simulink model "r2_c8n856m1k_vacc"  (R2013a)
settings: Solver=FixedStepDiscrete  SolverMode=SingleTasking  StartTime=0.0  StopTime=10000  RelTol=1e-3
undefined symbols (not defined in the file): Down, Up
libraries: xbsIndex_r4 x32309, casper_library_misc x83, casper_library_delays x13, casper_library_communications x8, xbsReplacements_r4 x3, xps_library x2
(part 51 of 251)

== system r2_c8n856m1k_vacc/pfb/pfb_fir_generic/pfb_fir_taps/ddata0 ==
  din : Inport
  en : Inport  Port=2
  del{1..96} (96 alike) : xbsIndex_r4/Delay  rst=off  infoeditControl=Selection of Reset will increase slice count due to use of real FFs and instead of SRLs  en=on  latency=2  dbl_ovrd=off  reg_retiming=on  block_type=delay
  din_expand : Subsystem  AncestorBlock=casper_library_flow_control/bus_expand  mask(bus_expand){mode=divisions of arbitrary size, outputNum=1, outputWidth=[10  10  10  10  10  10  10  10  10  10  10  10  10  10  10  10  10  10  10  10  10  10  10  10  10  10  10  10  10  10  10  10  10  10  10  10  10  10  10  10  10  10  10  10  10  10  10  10  10  10  10  10  10  10  10  10  10  10  10  10  10  10  10  10  10  10  10  10  10  10  10  10  10  10  10  10  10  10  10  10  10  10  10  10  10  10  10  10  10  10  10  10  10  10  10  10], outputBinaryPt=[0  0  0  0  0  0  0  0  0  0  0  0  0  0  0  0  0  0  0  0  0  0  0  0  0  0  0  0  0  0  0  0  0  0  0  0  0  0  0  0  0  0  0  0  0  0  0  0  0  0  0  0  0  0  0  0  0  0  0  0  0  0  0  0  0  0  0  0  0  0  0  0  0  0  0  0  0  0  0  0  0  0  0  0  0  0  0  0  0  0  0  0  0  0  0  0], outputArithmeticType=[0  0  0  0  0  0  0  0  0  0  0  0  0  0  0  0  0  0  0  0  0  0  0  0  0  0  0  0  0  0  0  0  0  0  0  0  0  0  0  0  0  0  0  0  0  0  0  0  0  0  0  0  0  0  0  0  0  0  0  0  0  0  0  0  0  0  0  0  0  0  0  0  0  0  0  0  0  0  0  0  0  0  0  0  0  0  0  0  0  0  0  0  0  0  0  0], show_format=on, outputToWorkspace=off, variablePrefix=, outputToModelAsWell=on}
      mask prompts: outputNum "Number of outputs:"; outputWidth "Output width [msb ... lsb]:"; outputBinaryPt "Output binary point position [msb ... lsb]:"; outputArithmeticType "Output arithmetic type (ufix=0, fix=1, bool=2) [msb ... lsb]:"; show_format "Print format string?"; outputToWorkspace "Output to workspace?"; variablePrefix "Variable name prefix:"; outputToModelAsWell "Output to model as well?"
      mask help:
      | Expands a 'bus' made using a bus_create or Xilinx
      | concat block.
      | The input will be divided into the specified number
      | of outputs with the specified arithmetic types.
      | Divisions are made from most significant bit.
      mask init: same as r2_c8n856m1k_vacc/bus_expand15
      settings: AttributesFormatString=96 outputs: uf10.0,uf10.0,uf10.0,uf10.0,uf10.0,uf10.0,uf10.0,uf10.0,uf10.0,uf10.0,uf10.0,uf10.0,uf10.0,uf10.0,uf10.0,uf10.0,uf10.0,uf10.0,uf10.0,uf10.0,uf10.0,uf10.0,uf10.0,uf10.0,uf10.0,uf10.0,uf10.0,uf10.0,uf10.0,uf10.0,uf10.0,uf10.0,uf10.0,uf10.0,uf10.0,uf10.0,uf10.0,uf10.0,uf10.0,uf10.0,uf10.0,uf10.0,uf10.0,uf10.0,uf10.0,uf10.0,uf10.0,uf10.0,uf10.0,uf10.0,uf10.0,uf10.0,uf10.0,uf10.0,uf10.0,uf10.0,uf10.0,uf10.0,uf10.0,uf10.0,uf10.0,uf10.0,uf10.0,uf10.0,uf10.0,uf10.0,uf10.0,uf10.0,uf10.0,uf10.0,uf10.0,uf10.0,uf10.0,uf10.0,uf10.0,uf10.0,uf10.0,uf10.0,uf10.0,uf10.0,uf10.0,uf10.0,uf10.0,uf10.0,uf10.0,uf10.0,uf10.0,uf10.0,uf10.0,uf10.0,uf10.0,uf10.0,uf10.0,uf10.0,uf10.0,uf10.0,
      mask TypeOptions mode: divisions of equal size | divisions of arbitrary size
      mask Callback mode: bus_expand_callback();
      mask Callback outputWidth: bus_expand_callback();
      mask Callback outputToWorkspace: bus_expand_callback();
  dout_compress : Subsystem  AncestorBlock=casper_library_flow_control/bus_create  mask(bus_create){inputNum=96}
      mask prompts: inputNum "Number of inputs:"
      mask init:
      | bus_create_init(gcb,...
      |     'inputNum', inputNum);
  en_expand : Subsystem  AncestorBlock=casper_library_flow_control/bus_expand  mask(bus_expand){mode=divisions of equal size, outputNum=96, outputWidth=1, outputBinaryPt=0, outputArithmeticType=2, show_format=on, outputToWorkspace=off, variablePrefix=, outputToModelAsWell=on}
      mask prompts: outputNum "Number of outputs:"; outputWidth "Output width:"; outputBinaryPt "Output binary point position:"; outputArithmeticType "Output arithmetic type (ufix=0, fix=1, bool=2):"; show_format "Print format string?"; outputToWorkspace "Output to workspace?"; variablePrefix "Variable name prefix:"; outputToModelAsWell "Output to model as well?"
      mask help:
      | Expands a 'bus' made using a bus_create or Xilinx
      | concat block.
      | The input will be divided into the specified number
      | of outputs with the specified arithmetic types.
      | Divisions are made from most significant bit.
      mask init: same as r2_c8n856m1k_vacc/bus_expand15
      settings: AttributesFormatString=96 outputs: b,b,b,b,b,b,b,b,b,b,b,b,b,b,b,b,b,b,b,b,b,b,b,b,b,b,b,b,b,b,b,b,b,b,b,b,b,b,b,b,b,b,b,b,b,b,b,b,b,b,b,b,b,b,b,b,b,b,b,b,b,b,b,b,b,b,b,b,b,b,b,b,b,b,b,b,b,b,b,b,b,b,b,b,b,b,b,b,b,b,b,b,b,b,b,b,
      mask TypeOptions mode: divisions of equal size | divisions of arbitrary size
      mask Callback mode: bus_expand_callback();
      mask Callback outputWidth: bus_expand_callback();
      mask Callback outputToWorkspace: bus_expand_callback();
  dout : Outport
  wires:
  din/1 -> din_expand/1(bus_in)
  en/1 -> en_expand/1(bus_in)
  din_expand/1(msb_out96) -> del1/1
  en_expand/1(msb_out96) -> del1/2
  din_expand/2(out95) -> del2/1
  en_expand/2(out95) -> del2/2
  din_expand/3(out94) -> del3/1
  en_expand/3(out94) -> del3/2
  din_expand/4(out93) -> del4/1
  en_expand/4(out93) -> del4/2
  din_expand/5(out92) -> del5/1
  en_expand/5(out92) -> del5/2
  din_expand/6(out91) -> del6/1
  en_expand/6(out91) -> del6/2
  din_expand/7(out90) -> del7/1
  en_expand/7(out90) -> del7/2
  din_expand/8(out89) -> del8/1
  en_expand/8(out89) -> del8/2
  din_expand/9(out88) -> del9/1
  en_expand/9(out88) -> del9/2
  din_expand/10(out87) -> del10/1
  en_expand/10(out87) -> del10/2
  din_expand/11(out86) -> del11/1
  en_expand/11(out86) -> del11/2
  din_expand/12(out85) -> del12/1
  en_expand/12(out85) -> del12/2
  din_expand/13(out84) -> del13/1
  en_expand/13(out84) -> del13/2
  din_expand/14(out83) -> del14/1
  en_expand/14(out83) -> del14/2
  din_expand/15(out82) -> del15/1
  en_expand/15(out82) -> del15/2
  din_expand/16(out81) -> del16/1
  en_expand/16(out81) -> del16/2
  din_expand/17(out80) -> del17/1
  en_expand/17(out80) -> del17/2
  din_expand/18(out79) -> del18/1
  en_expand/18(out79) -> del18/2
  din_expand/19(out78) -> del19/1
  en_expand/19(out78) -> del19/2
  din_expand/20(out77) -> del20/1
  en_expand/20(out77) -> del20/2
  din_expand/21(out76) -> del21/1
  en_expand/21(out76) -> del21/2
  din_expand/22(out75) -> del22/1
  en_expand/22(out75) -> del22/2
  din_expand/23(out74) -> del23/1
  en_expand/23(out74) -> del23/2
  din_expand/24(out73) -> del24/1
  en_expand/24(out73) -> del24/2
  din_expand/25(out72) -> del25/1
  en_expand/25(out72) -> del25/2
  din_expand/26(out71) -> del26/1
  en_expand/26(out71) -> del26/2
  din_expand/27(out70) -> del27/1
  en_expand/27(out70) -> del27/2
  din_expand/28(out69) -> del28/1
  en_expand/28(out69) -> del28/2
  din_expand/29(out68) -> del29/1
  en_expand/29(out68) -> del29/2
  din_expand/30(out67) -> del30/1
  en_expand/30(out67) -> del30/2
  din_expand/31(out66) -> del31/1
  en_expand/31(out66) -> del31/2
  din_expand/32(out65) -> del32/1
  en_expand/32(out65) -> del32/2
  din_expand/33(out64) -> del33/1
  en_expand/33(out64) -> del33/2
  din_expand/34(out63) -> del34/1
  en_expand/34(out63) -> del34/2
  din_expand/35(out62) -> del35/1
  en_expand/35(out62) -> del35/2
  din_expand/36(out61) -> del36/1
  en_expand/36(out61) -> del36/2
  din_expand/37(out60) -> del37/1
  en_expand/37(out60) -> del37/2
  din_expand/38(out59) -> del38/1
  en_expand/38(out59) -> del38/2
  din_expand/39(out58) -> del39/1
  en_expand/39(out58) -> del39/2
  din_expand/40(out57) -> del40/1
  en_expand/40(out57) -> del40/2
  din_expand/41(out56) -> del41/1
  en_expand/41(out56) -> del41/2
  din_expand/42(out55) -> del42/1
  en_expand/42(out55) -> del42/2
  din_expand/43(out54) -> del43/1
  en_expand/43(out54) -> del43/2
  din_expand/44(out53) -> del44/1
  en_expand/44(out53) -> del44/2
  din_expand/45(out52) -> del45/1
  en_expand/45(out52) -> del45/2
  din_expand/46(out51) -> del46/1
  en_expand/46(out51) -> del46/2
  din_expand/47(out50) -> del47/1
  en_expand/47(out50) -> del47/2
  din_expand/48(out49) -> del48/1
  en_expand/48(out49) -> del48/2
  din_expand/49(out48) -> del49/1
  en_expand/49(out48) -> del49/2
  din_expand/50(out47) -> del50/1
  en_expand/50(out47) -> del50/2
  din_expand/51(out46) -> del51/1
  en_expand/51(out46) -> del51/2
  din_expand/52(out45) -> del52/1
  en_expand/52(out45) -> del52/2
  din_expand/53(out44) -> del53/1
  en_expand/53(out44) -> del53/2
  din_expand/54(out43) -> del54/1
  en_expand/54(out43) -> del54/2
  din_expand/55(out42) -> del55/1
  en_expand/55(out42) -> del55/2
  din_expand/56(out41) -> del56/1
  en_expand/56(out41) -> del56/2
  din_expand/57(out40) -> del57/1
  en_expand/57(out40) -> del57/2
  din_expand/58(out39) -> del58/1
  en_expand/58(out39) -> del58/2
  din_expand/59(out38) -> del59/1
  en_expand/59(out38) -> del59/2
  din_expand/60(out37) -> del60/1
  en_expand/60(out37) -> del60/2
  din_expand/61(out36) -> del61/1
  en_expand/61(out36) -> del61/2
  din_expand/62(out35) -> del62/1
  en_expand/62(out35) -> del62/2
  din_expand/63(out34) -> del63/1
  en_expand/63(out34) -> del63/2
  din_expand/64(out33) -> del64/1
  en_expand/64(out33) -> del64/2
  din_expand/65(out32) -> del65/1
  en_expand/65(out32) -> del65/2
  din_expand/66(out31) -> del66/1
  en_expand/66(out31) -> del66/2
  din_expand/67(out30) -> del67/1
  en_expand/67(out30) -> del67/2
  din_expand/68(out29) -> del68/1
  en_expand/68(out29) -> del68/2
  din_expand/69(out28) -> del69/1
  en_expand/69(out28) -> del69/2
  din_expand/70(out27) -> del70/1
  en_expand/70(out27) -> del70/2
  din_expand/71(out26) -> del71/1
  en_expand/71(out26) -> del71/2
  din_expand/72(out25) -> del72/1
  en_expand/72(out25) -> del72/2
  din_expand/73(out24) -> del73/1
  en_expand/73(out24) -> del73/2
  din_expand/74(out23) -> del74/1
  en_expand/74(out23) -> del74/2
  din_expand/75(out22) -> del75/1
  en_expand/75(out22) -> del75/2
  din_expand/76(out21) -> del76/1
  en_expand/76(out21) -> del76/2
  din_expand/77(out20) -> del77/1
  en_expand/77(out20) -> del77/2
  din_expand/78(out19) -> del78/1
  en_expand/78(out19) -> del78/2
  din_expand/79(out18) -> del79/1
  en_expand/79(out18) -> del79/2
  din_expand/80(out17) -> del80/1
  en_expand/80(out17) -> del80/2
  din_expand/81(out16) -> del81/1
  en_expand/81(out16) -> del81/2
  din_expand/82(out15) -> del82/1
  en_expand/82(out15) -> del82/2
  din_expand/83(out14) -> del83/1
  en_expand/83(out14) -> del83/2
  din_expand/84(out13) -> del84/1
  en_expand/84(out13) -> del84/2
  din_expand/85(out12) -> del85/1
  en_expand/85(out12) -> del85/2
  din_expand/86(out11) -> del86/1
  en_expand/86(out11) -> del86/2
  din_expand/87(out10) -> del87/1
  en_expand/87(out10) -> del87/2
  din_expand/88(out9) -> del88/1
  en_expand/88(out9) -> del88/2
  din_expand/89(out8) -> del89/1
  en_expand/89(out8) -> del89/2
  din_expand/90(out7) -> del90/1
  en_expand/90(out7) -> del90/2
  din_expand/91(out6) -> del91/1
  en_expand/91(out6) -> del91/2
  din_expand/92(out5) -> del92/1
  en_expand/92(out5) -> del92/2
  din_expand/93(out4) -> del93/1
  en_expand/93(out4) -> del93/2
  din_expand/94(out3) -> del94/1
  en_expand/94(out3) -> del94/2
  din_expand/95(out2) -> del95/1
  en_expand/95(out2) -> del95/2
  din_expand/96(lsb_out1) -> del96/1
  en_expand/96(lsb_out1) -> del96/2
  del1/1 -> dout_compress/1(in1)
  del2/1 -> dout_compress/2(in2)
  del3/1 -> dout_compress/3(in3)
  del4/1 -> dout_compress/4(in4)
  del5/1 -> dout_compress/5(in5)
  del6/1 -> dout_compress/6(in6)
  del7/1 -> dout_compress/7(in7)
  del8/1 -> dout_compress/8(in8)
  del9/1 -> dout_compress/9(in9)
  del10/1 -> dout_compress/10(in10)
  del11/1 -> dout_compress/11(in11)
  del12/1 -> dout_compress/12(in12)
  del13/1 -> dout_compress/13(in13)
  del14/1 -> dout_compress/14(in14)
  del15/1 -> dout_compress/15(in15)
  del16/1 -> dout_compress/16(in16)
  del17/1 -> dout_compress/17(in17)
  del18/1 -> dout_compress/18(in18)
  del19/1 -> dout_compress/19(in19)
  del20/1 -> dout_compress/20(in20)
  del21/1 -> dout_compress/21(in21)
  del22/1 -> dout_compress/22(in22)
  del23/1 -> dout_compress/23(in23)
  del24/1 -> dout_compress/24(in24)
  del25/1 -> dout_compress/25(in25)
  del26/1 -> dout_compress/26(in26)
  del27/1 -> dout_compress/27(in27)
  del28/1 -> dout_compress/28(in28)
  del29/1 -> dout_compress/29(in29)
  del30/1 -> dout_compress/30(in30)
  del31/1 -> dout_compress/31(in31)
  del32/1 -> dout_compress/32(in32)
  del33/1 -> dout_compress/33(in33)
  del34/1 -> dout_compress/34(in34)
  del35/1 -> dout_compress/35(in35)
  del36/1 -> dout_compress/36(in36)
  del37/1 -> dout_compress/37(in37)
  del38/1 -> dout_compress/38(in38)
  del39/1 -> dout_compress/39(in39)
  del40/1 -> dout_compress/40(in40)
  del41/1 -> dout_compress/41(in41)
  del42/1 -> dout_compress/42(in42)
  del43/1 -> dout_compress/43(in43)
  del44/1 -> dout_compress/44(in44)
  del45/1 -> dout_compress/45(in45)
  del46/1 -> dout_compress/46(in46)
  del47/1 -> dout_compress/47(in47)
  del48/1 -> dout_compress/48(in48)
  del49/1 -> dout_compress/49(in49)
  del50/1 -> dout_compress/50(in50)
  del51/1 -> dout_compress/51(in51)
  del52/1 -> dout_compress/52(in52)
  del53/1 -> dout_compress/53(in53)
  del54/1 -> dout_compress/54(in54)
  del55/1 -> dout_compress/55(in55)
  del56/1 -> dout_compress/56(in56)
  del57/1 -> dout_compress/57(in57)
  del58/1 -> dout_compress/58(in58)
  del59/1 -> dout_compress/59(in59)
  del60/1 -> dout_compress/60(in60)
  del61/1 -> dout_compress/61(in61)
  del62/1 -> dout_compress/62(in62)
  del63/1 -> dout_compress/63(in63)
  del64/1 -> dout_compress/64(in64)
  del65/1 -> dout_compress/65(in65)
  del66/1 -> dout_compress/66(in66)
  del67/1 -> dout_compress/67(in67)
  del68/1 -> dout_compress/68(in68)
  del69/1 -> dout_compress/69(in69)
  del70/1 -> dout_compress/70(in70)
  del71/1 -> dout_compress/71(in71)
  del72/1 -> dout_compress/72(in72)
  del73/1 -> dout_compress/73(in73)
  del74/1 -> dout_compress/74(in74)
  del75/1 -> dout_compress/75(in75)
  del76/1 -> dout_compress/76(in76)
  del77/1 -> dout_compress/77(in77)
  del78/1 -> dout_compress/78(in78)
  del79/1 -> dout_compress/79(in79)
  del80/1 -> dout_compress/80(in80)
  del81/1 -> dout_compress/81(in81)
  del82/1 -> dout_compress/82(in82)
  del83/1 -> dout_compress/83(in83)
  del84/1 -> dout_compress/84(in84)
  del85/1 -> dout_compress/85(in85)
  del86/1 -> dout_compress/86(in86)
  del87/1 -> dout_compress/87(in87)
  del88/1 -> dout_compress/88(in88)
  del89/1 -> dout_compress/89(in89)
  del90/1 -> dout_compress/90(in90)
  del91/1 -> dout_compress/91(in91)
  del92/1 -> dout_compress/92(in92)
  del93/1 -> dout_compress/93(in93)
  del94/1 -> dout_compress/94(in94)
  del95/1 -> dout_compress/95(in95)
  del96/1 -> dout_compress/96(in96)
  dout_compress/1(bus_out) -> dout/1

== system r2_c8n856m1k_vacc/pfb/pfb_fir_generic/pfb_fir_taps/ddata1 ==
  din : Inport
  en : Inport  Port=2
  del{1..112} (112 alike) : xbsIndex_r4/Delay  rst=off  infoeditControl=Selection of Reset will increase slice count due to use of real FFs and instead of SRLs  en=on  latency=2  dbl_ovrd=off  reg_retiming=on  block_type=delay
  din_expand : Subsystem  AncestorBlock=casper_library_flow_control/bus_expand  mask(bus_expand){mode=divisions of arbitrary size, outputNum=1, outputWidth=[10  10  10  10  10  10  10  10  10  10  10  10  10  10  10  10  10  10  10  10  10  10  10  10  10  10  10  10  10  10  10  10  10  10  10  10  10  10  10  10  10  10  10  10  10  10  10  10  10  10  10  10  10  10  10  10  10  10  10  10  10  10  10  10  10  10  10  10  10  10  10  10  10  10  10  10  10  10  10  10  10  10  10  10  10  10  10  10  10  10  10  10  10  10  10  10  10  10  10  10  10  10  10  10  10  10  10  10  10  10  10  10], outputBinaryPt=[0  0  0  0  0  0  0  0  0  0  0  0  0  0  0  0  0  0  0  0  0  0  0  0  0  0  0  0  0  0  0  0  0  0  0  0  0  0  0  0  0  0  0  0  0  0  0  0  0  0  0  0  0  0  0  0  0  0  0  0  0  0  0  0  0  0  0  0  0  0  0  0  0  0  0  0  0  0  0  0  0  0  0  0  0  0  0  0  0  0  0  0  0  0  0  0  0  0  0  0  0  0  0  0  0  0  0  0  0  0  0  0], outputArithmeticType=[0  0  0  0  0  0  0  0  0  0  0  0  0  0  0  0  0  0  0  0  0  0  0  0  0  0  0  0  0  0  0  0  0  0  0  0  0  0  0  0  0  0  0  0  0  0  0  0  0  0  0  0  0  0  0  0  0  0  0  0  0  0  0  0  0  0  0  0  0  0  0  0  0  0  0  0  0  0  0  0  0  0  0  0  0  0  0  0  0  0  0  0  0  0  0  0  0  0  0  0  0  0  0  0  0  0  0  0  0  0  0  0], show_format=on, outputToWorkspace=off, variablePrefix=, outputToModelAsWell=on}
      mask prompts: outputNum "Number of outputs:"; outputWidth "Output width [msb ... lsb]:"; outputBinaryPt "Output binary point position [msb ... lsb]:"; outputArithmeticType "Output arithmetic type (ufix=0, fix=1, bool=2) [msb ... lsb]:"; show_format "Print format string?"; outputToWorkspace "Output to workspace?"; variablePrefix "Variable name prefix:"; outputToModelAsWell "Output to model as well?"
      mask help:
      | Expands a 'bus' made using a bus_create or Xilinx
      | concat block.
      | The input will be divided into the specified number
      | of outputs with the specified arithmetic types.
      | Divisions are made from most significant bit.
      mask init: same as r2_c8n856m1k_vacc/bus_expand15
      settings: AttributesFormatString=112 outputs: uf10.0,uf10.0,uf10.0,uf10.0,uf10.0,uf10.0,uf10.0,uf10.0,uf10.0,uf10.0,uf10.0,uf10.0,uf10.0,uf10.0,uf10.0,uf10.0,uf10.0,uf10.0,uf10.0,uf10.0,uf10.0,uf10.0,uf10.0,uf10.0,uf10.0,uf10.0,uf10.0,uf10.0,uf10.0,uf10.0,uf10.0,uf10.0,uf10.0,uf10.0,uf10.0,uf10.0,uf10.0,uf10.0,uf10.0,uf10.0,uf10.0,uf10.0,uf10.0,uf10.0,uf10.0,uf10.0,uf10.0,uf10.0,uf10.0,uf10.0,uf10.0,uf10.0,uf10.0,uf10.0,uf10.0,uf10.0,uf10.0,uf10.0,uf10.0,uf10.0,uf10.0,uf10.0,uf10.0,uf10.0,uf10.0,uf10.0,uf10.0,uf10.0,uf10.0,uf10.0,uf10.0,uf10.0,uf10.0,uf10.0,uf10.0,uf10.0,uf10.0,uf10.0,uf10.0,uf10.0,uf10.0,uf10.0,uf10.0,uf10.0,uf10.0,uf10.0,uf10.0,uf10.0,uf10.0,uf10.0,uf10.0,uf10.0,uf10.0,uf10.0,uf10.0,uf10.0,uf10.0,uf10.0,uf10.0,uf10.0,uf10.0,uf10.0,uf10.0,uf10.0,uf10.0,uf10.0,uf10.0,uf10.0,uf10.0,uf10.0,uf10.0,uf10.0,
      mask TypeOptions mode: divisions of equal size | divisions of arbitrary size
      mask Callback mode: bus_expand_callback();
      mask Callback outputWidth: bus_expand_callback();
      mask Callback outputToWorkspace: bus_expand_callback();
  dout_compress : Subsystem  AncestorBlock=casper_library_flow_control/bus_create  mask(bus_create){inputNum=112}
      mask prompts: inputNum "Number of inputs:"
      mask init:
      | bus_create_init(gcb,...
      |     'inputNum', inputNum);
  en_expand : Subsystem  AncestorBlock=casper_library_flow_control/bus_expand  mask(bus_expand){mode=divisions of equal size, outputNum=112, outputWidth=1, outputBinaryPt=0, outputArithmeticType=2, show_format=on, outputToWorkspace=off, variablePrefix=, outputToModelAsWell=on}
      mask prompts: outputNum "Number of outputs:"; outputWidth "Output width:"; outputBinaryPt "Output binary point position:"; outputArithmeticType "Output arithmetic type (ufix=0, fix=1, bool=2):"; show_format "Print format string?"; outputToWorkspace "Output to workspace?"; variablePrefix "Variable name prefix:"; outputToModelAsWell "Output to model as well?"
      mask help:
      | Expands a 'bus' made using a bus_create or Xilinx
      | concat block.
      | The input will be divided into the specified number
      | of outputs with the specified arithmetic types.
      | Divisions are made from most significant bit.
      mask init: same as r2_c8n856m1k_vacc/bus_expand15
      settings: AttributesFormatString=112 outputs: b,b,b,b,b,b,b,b,b,b,b,b,b,b,b,b,b,b,b,b,b,b,b,b,b,b,b,b,b,b,b,b,b,b,b,b,b,b,b,b,b,b,b,b,b,b,b,b,b,b,b,b,b,b,b,b,b,b,b,b,b,b,b,b,b,b,b,b,b,b,b,b,b,b,b,b,b,b,b,b,b,b,b,b,b,b,b,b,b,b,b,b,b,b,b,b,b,b,b,b,b,b,b,b,b,b,b,b,b,b,b,b,
      mask TypeOptions mode: divisions of equal size | divisions of arbitrary size
      mask Callback mode: bus_expand_callback();
      mask Callback outputWidth: bus_expand_callback();
      mask Callback outputToWorkspace: bus_expand_callback();
  dout : Outport
  wires:
  din/1 -> din_expand/1(bus_in)
  en/1 -> en_expand/1(bus_in)
  din_expand/1(msb_out112) -> del1/1
  en_expand/1(msb_out112) -> del1/2
  din_expand/2(out111) -> del2/1
  en_expand/2(out111) -> del2/2
  din_expand/3(out110) -> del3/1
  en_expand/3(out110) -> del3/2
  din_expand/4(out109) -> del4/1
  en_expand/4(out109) -> del4/2
  din_expand/5(out108) -> del5/1
  en_expand/5(out108) -> del5/2
  din_expand/6(out107) -> del6/1
  en_expand/6(out107) -> del6/2
  din_expand/7(out106) -> del7/1
  en_expand/7(out106) -> del7/2
  din_expand/8(out105) -> del8/1
  en_expand/8(out105) -> del8/2
  din_expand/9(out104) -> del9/1
  en_expand/9(out104) -> del9/2
  din_expand/10(out103) -> del10/1
  en_expand/10(out103) -> del10/2
  din_expand/11(out102) -> del11/1
  en_expand/11(out102) -> del11/2
  din_expand/12(out101) -> del12/1
  en_expand/12(out101) -> del12/2
  din_expand/13(out100) -> del13/1
  en_expand/13(out100) -> del13/2
  din_expand/14(out99) -> del14/1
  en_expand/14(out99) -> del14/2
  din_expand/15(out98) -> del15/1
  en_expand/15(out98) -> del15/2
  din_expand/16(out97) -> del16/1
  en_expand/16(out97) -> del16/2
  din_expand/17(out96) -> del17/1
  en_expand/17(out96) -> del17/2
  din_expand/18(out95) -> del18/1
  en_expand/18(out95) -> del18/2
  din_expand/19(out94) -> del19/1
  en_expand/19(out94) -> del19/2
  din_expand/20(out93) -> del20/1
  en_expand/20(out93) -> del20/2
  din_expand/21(out92) -> del21/1
  en_expand/21(out92) -> del21/2
  din_expand/22(out91) -> del22/1
  en_expand/22(out91) -> del22/2
  din_expand/23(out90) -> del23/1
  en_expand/23(out90) -> del23/2
  din_expand/24(out89) -> del24/1
  en_expand/24(out89) -> del24/2
  din_expand/25(out88) -> del25/1
  en_expand/25(out88) -> del25/2
  din_expand/26(out87) -> del26/1
  en_expand/26(out87) -> del26/2
  din_expand/27(out86) -> del27/1
  en_expand/27(out86) -> del27/2
  din_expand/28(out85) -> del28/1
  en_expand/28(out85) -> del28/2
  din_expand/29(out84) -> del29/1
  en_expand/29(out84) -> del29/2
  din_expand/30(out83) -> del30/1
  en_expand/30(out83) -> del30/2
  din_expand/31(out82) -> del31/1
  en_expand/31(out82) -> del31/2
  din_expand/32(out81) -> del32/1
  en_expand/32(out81) -> del32/2
  din_expand/33(out80) -> del33/1
  en_expand/33(out80) -> del33/2
  din_expand/34(out79) -> del34/1
  en_expand/34(out79) -> del34/2
  din_expand/35(out78) -> del35/1
  en_expand/35(out78) -> del35/2
  din_expand/36(out77) -> del36/1
  en_expand/36(out77) -> del36/2
  din_expand/37(out76) -> del37/1
  en_expand/37(out76) -> del37/2
  din_expand/38(out75) -> del38/1
  en_expand/38(out75) -> del38/2
  din_expand/39(out74) -> del39/1
  en_expand/39(out74) -> del39/2
  din_expand/40(out73) -> del40/1
  en_expand/40(out73) -> del40/2
  din_expand/41(out72) -> del41/1
  en_expand/41(out72) -> del41/2
  din_expand/42(out71) -> del42/1
  en_expand/42(out71) -> del42/2
  din_expand/43(out70) -> del43/1
  en_expand/43(out70) -> del43/2
  din_expand/44(out69) -> del44/1
  en_expand/44(out69) -> del44/2
  din_expand/45(out68) -> del45/1
  en_expand/45(out68) -> del45/2
  din_expand/46(out67) -> del46/1
  en_expand/46(out67) -> del46/2
  din_expand/47(out66) -> del47/1
  en_expand/47(out66) -> del47/2
  din_expand/48(out65) -> del48/1
  en_expand/48(out65) -> del48/2
  din_expand/49(out64) -> del49/1
  en_expand/49(out64) -> del49/2
  din_expand/50(out63) -> del50/1
  en_expand/50(out63) -> del50/2
  din_expand/51(out62) -> del51/1
  en_expand/51(out62) -> del51/2
  din_expand/52(out61) -> del52/1
  en_expand/52(out61) -> del52/2
  din_expand/53(out60) -> del53/1
  en_expand/53(out60) -> del53/2
  din_expand/54(out59) -> del54/1
  en_expand/54(out59) -> del54/2
  din_expand/55(out58) -> del55/1
  en_expand/55(out58) -> del55/2
  din_expand/56(out57) -> del56/1
  en_expand/56(out57) -> del56/2
  din_expand/57(out56) -> del57/1
  en_expand/57(out56) -> del57/2
  din_expand/58(out55) -> del58/1
  en_expand/58(out55) -> del58/2
  din_expand/59(out54) -> del59/1
  en_expand/59(out54) -> del59/2
  din_expand/60(out53) -> del60/1
  en_expand/60(out53) -> del60/2
  din_expand/61(out52) -> del61/1
  en_expand/61(out52) -> del61/2
  din_expand/62(out51) -> del62/1
  en_expand/62(out51) -> del62/2
  din_expand/63(out50) -> del63/1
  en_expand/63(out50) -> del63/2
  din_expand/64(out49) -> del64/1
  en_expand/64(out49) -> del64/2
  din_expand/65(out48) -> del65/1
  en_expand/65(out48) -> del65/2
  din_expand/66(out47) -> del66/1
  en_expand/66(out47) -> del66/2
  din_expand/67(out46) -> del67/1
  en_expand/67(out46) -> del67/2
  din_expand/68(out45) -> del68/1
  en_expand/68(out45) -> del68/2
  din_expand/69(out44) -> del69/1
  en_expand/69(out44) -> del69/2
  din_expand/70(out43) -> del70/1
  en_expand/70(out43) -> del70/2
  din_expand/71(out42) -> del71/1
  en_expand/71(out42) -> del71/2
  din_expand/72(out41) -> del72/1
  en_expand/72(out41) -> del72/2
  din_expand/73(out40) -> del73/1
  en_expand/73(out40) -> del73/2
  din_expand/74(out39) -> del74/1
  en_expand/74(out39) -> del74/2
  din_expand/75(out38) -> del75/1
  en_expand/75(out38) -> del75/2
  din_expand/76(out37) -> del76/1
  en_expand/76(out37) -> del76/2
  din_expand/77(out36) -> del77/1
  en_expand/77(out36) -> del77/2
  din_expand/78(out35) -> del78/1
  en_expand/78(out35) -> del78/2
  din_expand/79(out34) -> del79/1
  en_expand/79(out34) -> del79/2
  din_expand/80(out33) -> del80/1
  en_expand/80(out33) -> del80/2
  din_expand/81(out32) -> del81/1
  en_expand/81(out32) -> del81/2
  din_expand/82(out31) -> del82/1
  en_expand/82(out31) -> del82/2
  din_expand/83(out30) -> del83/1
  en_expand/83(out30) -> del83/2
  din_expand/84(out29) -> del84/1
  en_expand/84(out29) -> del84/2
  din_expand/85(out28) -> del85/1
  en_expand/85(out28) -> del85/2
  din_expand/86(out27) -> del86/1
  en_expand/86(out27) -> del86/2
  din_expand/87(out26) -> del87/1
  en_expand/87(out26) -> del87/2
  din_expand/88(out25) -> del88/1
  en_expand/88(out25) -> del88/2
  din_expand/89(out24) -> del89/1
  en_expand/89(out24) -> del89/2
  din_expand/90(out23) -> del90/1
  en_expand/90(out23) -> del90/2
  din_expand/91(out22) -> del91/1
  en_expand/91(out22) -> del91/2
  din_expand/92(out21) -> del92/1
  en_expand/92(out21) -> del92/2
  din_expand/93(out20) -> del93/1
  en_expand/93(out20) -> del93/2
  din_expand/94(out19) -> del94/1
  en_expand/94(out19) -> del94/2
  din_expand/95(out18) -> del95/1
  en_expand/95(out18) -> del95/2
  din_expand/96(out17) -> del96/1
  en_expand/96(out17) -> del96/2
  din_expand/97(out16) -> del97/1
  en_expand/97(out16) -> del97/2
  din_expand/98(out15) -> del98/1
  en_expand/98(out15) -> del98/2
  din_expand/99(out14) -> del99/1
  en_expand/99(out14) -> del99/2
  din_expand/100(out13) -> del100/1
  en_expand/100(out13) -> del100/2
  din_expand/101(out12) -> del101/1
  en_expand/101(out12) -> del101/2
  din_expand/102(out11) -> del102/1
  en_expand/102(out11) -> del102/2
  din_expand/103(out10) -> del103/1
  en_expand/103(out10) -> del103/2
  din_expand/104(out9) -> del104/1
  en_expand/104(out9) -> del104/2
  din_expand/105(out8) -> del105/1
  en_expand/105(out8) -> del105/2
  din_expand/106(out7) -> del106/1
  en_expand/106(out7) -> del106/2
  din_expand/107(out6) -> del107/1
  en_expand/107(out6) -> del107/2
  din_expand/108(out5) -> del108/1
  en_expand/108(out5) -> del108/2
  din_expand/109(out4) -> del109/1
  en_expand/109(out4) -> del109/2
  din_expand/110(out3) -> del110/1
  en_expand/110(out3) -> del110/2
  din_expand/111(out2) -> del111/1
  en_expand/111(out2) -> del111/2
  din_expand/112(lsb_out1) -> del112/1
  en_expand/112(lsb_out1) -> del112/2
  del1/1 -> dout_compress/1(in1)
  del2/1 -> dout_compress/2(in2)
  del3/1 -> dout_compress/3(in3)
  del4/1 -> dout_compress/4(in4)
  del5/1 -> dout_compress/5(in5)
  del6/1 -> dout_compress/6(in6)
  del7/1 -> dout_compress/7(in7)
  del8/1 -> dout_compress/8(in8)
  del9/1 -> dout_compress/9(in9)
  del10/1 -> dout_compress/10(in10)
  del11/1 -> dout_compress/11(in11)
  del12/1 -> dout_compress/12(in12)
  del13/1 -> dout_compress/13(in13)
  del14/1 -> dout_compress/14(in14)
  del15/1 -> dout_compress/15(in15)
  del16/1 -> dout_compress/16(in16)
  del17/1 -> dout_compress/17(in17)
  del18/1 -> dout_compress/18(in18)
  del19/1 -> dout_compress/19(in19)
  del20/1 -> dout_compress/20(in20)
  del21/1 -> dout_compress/21(in21)
  del22/1 -> dout_compress/22(in22)
  del23/1 -> dout_compress/23(in23)
  del24/1 -> dout_compress/24(in24)
  del25/1 -> dout_compress/25(in25)
  del26/1 -> dout_compress/26(in26)
  del27/1 -> dout_compress/27(in27)
  del28/1 -> dout_compress/28(in28)
  del29/1 -> dout_compress/29(in29)
  del30/1 -> dout_compress/30(in30)
  del31/1 -> dout_compress/31(in31)
  del32/1 -> dout_compress/32(in32)
  del33/1 -> dout_compress/33(in33)
  del34/1 -> dout_compress/34(in34)
  del35/1 -> dout_compress/35(in35)
  del36/1 -> dout_compress/36(in36)
  del37/1 -> dout_compress/37(in37)
  del38/1 -> dout_compress/38(in38)
  del39/1 -> dout_compress/39(in39)
  del40/1 -> dout_compress/40(in40)
  del41/1 -> dout_compress/41(in41)
  del42/1 -> dout_compress/42(in42)
  del43/1 -> dout_compress/43(in43)
  del44/1 -> dout_compress/44(in44)
  del45/1 -> dout_compress/45(in45)
  del46/1 -> dout_compress/46(in46)
  del47/1 -> dout_compress/47(in47)
  del48/1 -> dout_compress/48(in48)
  del49/1 -> dout_compress/49(in49)
  del50/1 -> dout_compress/50(in50)
  del51/1 -> dout_compress/51(in51)
  del52/1 -> dout_compress/52(in52)
  del53/1 -> dout_compress/53(in53)
  del54/1 -> dout_compress/54(in54)
  del55/1 -> dout_compress/55(in55)
  del56/1 -> dout_compress/56(in56)
  del57/1 -> dout_compress/57(in57)
  del58/1 -> dout_compress/58(in58)
  del59/1 -> dout_compress/59(in59)
  del60/1 -> dout_compress/60(in60)
  del61/1 -> dout_compress/61(in61)
  del62/1 -> dout_compress/62(in62)
  del63/1 -> dout_compress/63(in63)
  del64/1 -> dout_compress/64(in64)
  del65/1 -> dout_compress/65(in65)
  del66/1 -> dout_compress/66(in66)
  del67/1 -> dout_compress/67(in67)
  del68/1 -> dout_compress/68(in68)
  del69/1 -> dout_compress/69(in69)
  del70/1 -> dout_compress/70(in70)
  del71/1 -> dout_compress/71(in71)
  del72/1 -> dout_compress/72(in72)
  del73/1 -> dout_compress/73(in73)
  del74/1 -> dout_compress/74(in74)
  del75/1 -> dout_compress/75(in75)
  del76/1 -> dout_compress/76(in76)
  del77/1 -> dout_compress/77(in77)
  del78/1 -> dout_compress/78(in78)
  del79/1 -> dout_compress/79(in79)
  del80/1 -> dout_compress/80(in80)
  del81/1 -> dout_compress/81(in81)
  del82/1 -> dout_compress/82(in82)
  del83/1 -> dout_compress/83(in83)
  del84/1 -> dout_compress/84(in84)
  del85/1 -> dout_compress/85(in85)
  del86/1 -> dout_compress/86(in86)
  del87/1 -> dout_compress/87(in87)
  del88/1 -> dout_compress/88(in88)
  del89/1 -> dout_compress/89(in89)
  del90/1 -> dout_compress/90(in90)
  del91/1 -> dout_compress/91(in91)
  del92/1 -> dout_compress/92(in92)
  del93/1 -> dout_compress/93(in93)
  del94/1 -> dout_compress/94(in94)
  del95/1 -> dout_compress/95(in95)
  del96/1 -> dout_compress/96(in96)
  del97/1 -> dout_compress/97(in97)
  del98/1 -> dout_compress/98(in98)
  del99/1 -> dout_compress/99(in99)
  del100/1 -> dout_compress/100(in100)
  del101/1 -> dout_compress/101(in101)
  del102/1 -> dout_compress/102(in102)
  del103/1 -> dout_compress/103(in103)
  del104/1 -> dout_compress/104(in104)
  del105/1 -> dout_compress/105(in105)
  del106/1 -> dout_compress/106(in106)
  del107/1 -> dout_compress/107(in107)
  del108/1 -> dout_compress/108(in108)
  del109/1 -> dout_compress/109(in109)
  del110/1 -> dout_compress/110(in110)
  del111/1 -> dout_compress/111(in111)
  del112/1 -> dout_compress/112(in112)
  dout_compress/1(bus_out) -> dout/1

== system r2_c8n856m1k_vacc/pfb/pfb_fir_generic/pfb_fir_taps/delay_bram ==
  addr : Inport
  din : Inport  Port=2
  we : Inport  Port=3
  en : Inport  Port=4
  bram{0..31} (32 alike) : xbsIndex_r4/Single Port RAM  depth=256  initVector=getfield(get_param(gcb, 'UserData'), 'initVector')  distributed_mem=Block RAM  write_mode=Read Before Write  rst=off  init_reg=0  en=on  latency=9  dbl_ovrd=off  optimize=Speed  block_type=spram
  ddin : xbsIndex_r4/Delay  rst=off  infoeditControl=Selection of Reset will increase slice count due to use of real FFs and instead of SRLs  en=off  latency=0  dbl_ovrd=off  reg_retiming=on  block_type=delay
  debus_addr : Subsystem  AncestorBlock=casper_library_flow_control/bus_expand  mask(bus_expand){mode=divisions of equal size, outputNum=32, outputWidth=[8 8 8 8 8 8 8 8 8 8 8 8 8 8 8 8 8 8 8 8 8 8 8 8 8 8 8 8 8 8 8 8], outputBinaryPt=[0 0 0 0 0 0 0 0 0 0 0 0 0 0 0 0 0 0 0 0 0 0 0 0 0 0 0 0 0 0 0 0], outputArithmeticType=[0 0 0 0 0 0 0 0 0 0 0 0 0 0 0 0 0 0 0 0 0 0 0 0 0 0 0 0 0 0 0 0], show_format=on, outputToWorkspace=off, variablePrefix=, outputToModelAsWell=on}
      mask prompts: outputNum "Number of outputs:"; outputWidth "Output width:"; outputBinaryPt "Output binary point position:"; outputArithmeticType "Output arithmetic type (ufix=0, fix=1, bool=2):"; show_format "Print format string?"; outputToWorkspace "Output to workspace?"; variablePrefix "Variable name prefix:"; outputToModelAsWell "Output to model as well?"
      mask help:
      | Expands a 'bus' made using a bus_create or Xilinx
      | concat block.
      | The input will be divided into the specified number
      | of outputs with the specified arithmetic types.
      | Divisions are made from most significant bit.
      mask init: same as r2_c8n856m1k_vacc/bus_expand15
      settings: AttributesFormatString=32 outputs: uf8.0,uf8.0,uf8.0,uf8.0,uf8.0,uf8.0,uf8.0,uf8.0,uf8.0,uf8.0,uf8.0,uf8.0,uf8.0,uf8.0,uf8.0,uf8.0,uf8.0,uf8.0,uf8.0,uf8.0,uf8.0,uf8.0,uf8.0,uf8.0,uf8.0,uf8.0,uf8.0,uf8.0,uf8.0,uf8.0,uf8.0,uf8.0,
      mask TypeOptions mode: divisions of equal size | divisions of arbitrary size
      mask Callback mode: bus_expand_callback();
      mask Callback outputWidth: bus_expand_callback();
      mask Callback outputToWorkspace: bus_expand_callback();
  debus_din : Subsystem  AncestorBlock=casper_library_flow_control/bus_expand  mask(bus_expand){mode=divisions of arbitrary size, outputNum=1, outputWidth=[4 36 36 36 36 36 36 36 36 36 36 36 36 36 36 36 36 36 36 36 36 36 36 36 36 36 36 36 36 36 36 36], outputBinaryPt=[0 0 0 0 0 0 0 0 0 0 0 0 0 0 0 0 0 0 0 0 0 0 0 0 0 0 0 0 0 0 0 0], outputArithmeticType=[0 0 0 0 0 0 0 0 0 0 0 0 0 0 0 0 0 0 0 0 0 0 0 0 0 0 0 0 0 0 0 0], show_format=on, outputToWorkspace=off, variablePrefix=, outputToModelAsWell=on}
      mask prompts: outputNum "Number of outputs:"; outputWidth "Output width [msb ... lsb]:"; outputBinaryPt "Output binary point position [msb ... lsb]:"; outputArithmeticType "Output arithmetic type (ufix=0, fix=1, bool=2) [msb ... lsb]:"; show_format "Print format string?"; outputToWorkspace "Output to workspace?"; variablePrefix "Variable name prefix:"; outputToModelAsWell "Output to model as well?"
      mask help:
      | Expands a 'bus' made using a bus_create or Xilinx
      | concat block.
      | The input will be divided into the specified number
      | of outputs with the specified arithmetic types.
      | Divisions are made from most significant bit.
      mask init: same as r2_c8n856m1k_vacc/bus_expand15
      settings: AttributesFormatString=32 outputs: uf4.0,uf36.0,uf36.0,uf36.0,uf36.0,uf36.0,uf36.0,uf36.0,uf36.0,uf36.0,uf36.0,uf36.0,uf36.0,uf36.0,uf36.0,uf36.0,uf36.0,uf36.0,uf36.0,uf36.0,uf36.0,uf36.0,uf36.0,uf36.0,uf36.0,uf36.0,uf36.0,uf36.0,uf36.0,uf36.0,uf36.0,uf36.0,
      mask TypeOptions mode: divisions of equal size | divisions of arbitrary size
      mask Callback mode: bus_expand_callback();
      mask Callback outputWidth: bus_expand_callback();
      mask Callback outputToWorkspace: bus_expand_callback();
  debus_en : Subsystem  AncestorBlock=casper_library_flow_control/bus_expand  mask(bus_expand){mode=divisions of equal size, outputNum=32, outputWidth=[1 1 1 1 1 1 1 1 1 1 1 1 1 1 1 1 1 1 1 1 1 1 1 1 1 1 1 1 1 1 1 1], outputBinaryPt=[0 0 0 0 0 0 0 0 0 0 0 0 0 0 0 0 0 0 0 0 0 0 0 0 0 0 0 0 0 0 0 0], outputArithmeticType=[2 2 2 2 2 2 2 2 2 2 2 2 2 2 2 2 2 2 2 2 2 2 2 2 2 2 2 2 2 2 2 2], show_format=on, outputToWorkspace=off, variablePrefix=, outputToModelAsWell=on}
      mask prompts: outputNum "Number of outputs:"; outputWidth "Output width:"; outputBinaryPt "Output binary point position:"; outputArithmeticType "Output arithmetic type (ufix=0, fix=1, bool=2):"; show_format "Print format string?"; outputToWorkspace "Output to workspace?"; variablePrefix "Variable name prefix:"; outputToModelAsWell "Output to model as well?"
      mask help:
      | Expands a 'bus' made using a bus_create or Xilinx
      | concat block.
      | The input will be divided into the specified number
      | of outputs with the specified arithmetic types.
      | Divisions are made from most significant bit.
      mask init: same as r2_c8n856m1k_vacc/bus_expand15
      settings: AttributesFormatString=32 outputs: b,b,b,b,b,b,b,b,b,b,b,b,b,b,b,b,b,b,b,b,b,b,b,b,b,b,b,b,b,b,b,b,
      mask TypeOptions mode: divisions of equal size | divisions of arbitrary size
      mask Callback mode: bus_expand_callback();
      mask Callback outputWidth: bus_expand_callback();
      mask Callback outputToWorkspace: bus_expand_callback();
  debus_we : Subsystem  AncestorBlock=casper_library_flow_control/bus_expand  mask(bus_expand){mode=divisions of equal size, outputNum=32, outputWidth=[1 1 1 1 1 1 1 1 1 1 1 1 1 1 1 1 1 1 1 1 1 1 1 1 1 1 1 1 1 1 1 1], outputBinaryPt=[0 0 0 0 0 0 0 0 0 0 0 0 0 0 0 0 0 0 0 0 0 0 0 0 0 0 0 0 0 0 0 0], outputArithmeticType=[2 2 2 2 2 2 2 2 2 2 2 2 2 2 2 2 2 2 2 2 2 2 2 2 2 2 2 2 2 2 2 2], show_format=on, outputToWorkspace=off, variablePrefix=, outputToModelAsWell=on}
      mask prompts: outputNum "Number of outputs:"; outputWidth "Output width:"; outputBinaryPt "Output binary point position:"; outputArithmeticType "Output arithmetic type (ufix=0, fix=1, bool=2):"; show_format "Print format string?"; outputToWorkspace "Output to workspace?"; variablePrefix "Variable name prefix:"; outputToModelAsWell "Output to model as well?"
      mask help:
      | Expands a 'bus' made using a bus_create or Xilinx
      | concat block.
      | The input will be divided into the specified number
      | of outputs with the specified arithmetic types.
      | Divisions are made from most significant bit.
      mask init: same as r2_c8n856m1k_vacc/bus_expand15
      settings: AttributesFormatString=32 outputs: b,b,b,b,b,b,b,b,b,b,b,b,b,b,b,b,b,b,b,b,b,b,b,b,b,b,b,b,b,b,b,b,
      mask TypeOptions mode: divisions of equal size | divisions of arbitrary size
      mask Callback mode: bus_expand_callback();
      mask Callback outputWidth: bus_expand_callback();
      mask Callback outputToWorkspace: bus_expand_callback();
  den : xbsIndex_r4/Delay  rst=off  infoeditControl=Selection of Reset will increase slice count due to use of real FFs and instead of SRLs  en=off  latency=9  dbl_ovrd=off  reg_retiming=on  block_type=delay
  din_bussify : Subsystem  AncestorBlock=casper_library_flow_control/bus_create  mask(bus_create){inputNum=32}
      mask prompts: inputNum "Number of inputs:"
      mask init:
      | bus_create_init(gcb,...
      |     'inputNum', inputNum);
  rep_addr : Subsystem  AncestorBlock=casper_library_bus/bus_replicate  mask(bus_replicate){replication=32, latency=9, misc=off, implementation=core}
      mask prompts: replication "replication factor"; misc "misc support"; implementation "delay implementation"
      mask help: Output bus formed by replicating input bus a number of times
      mask init:
      | bus_replicate_init(gcb, ...
      |     'replication', replication, ...
      |     'latency', latency, ...
      |     'implementation', implementation, ...
      |     'misc', misc);
      mask TypeOptions implementation: core | behavioral
  rep_en : Subsystem  AncestorBlock=casper_library_bus/bus_replicate  mask(bus_replicate){replication=32, latency=9, misc=off, implementation=core}
      mask prompts: replication "replication factor"; misc "misc support"; implementation "delay implementation"
      mask help: Output bus formed by replicating input bus a number of times
      mask init:
      | bus_replicate_init(gcb, ...
      |     'replication', replication, ...
      |     'latency', latency, ...
      |     'implementation', implementation, ...
      |     'misc', misc);
      mask TypeOptions implementation: core | behavioral
  rep_we : Subsystem  AncestorBlock=casper_library_bus/bus_replicate  mask(bus_replicate){replication=32, latency=0, misc=off, implementation=behavioral}
      mask prompts: replication "replication factor"; misc "misc support"; implementation "delay implementation"
      mask help: Output bus formed by replicating input bus a number of times
      mask init:
      | bus_replicate_init(gcb, ...
      |     'replication', replication, ...
      |     'latency', latency, ...
      |     'implementation', implementation, ...
      |     'misc', misc);
      mask TypeOptions implementation: core | behavioral
  dout : Outport
  dvalid : Outport  Port=2
  wires:
  addr/1 -> rep_addr/1(in)
  din/1 -> ddin/1
  we/1 -> rep_we/1(in)
  en/1 -> rep_en/1(in)
  rep_addr/1(out) -> debus_addr/1(bus_in)
  ddin/1 -> debus_din/1(bus_in)
  rep_we/1(out) -> debus_we/1(bus_in)
  rep_en/1(out) -> debus_en/1(bus_in)
  debus_addr/1(msb_out32) -> bram0/1
  debus_din/1(msb_out32) -> bram0/2
  debus_we/1(msb_out32) -> bram0/3
  debus_en/1(msb_out32) -> bram0/4
  debus_addr/2(out31) -> bram1/1
  debus_din/2(out31) -> bram1/2
  debus_we/2(out31) -> bram1/3
  debus_en/2(out31) -> bram1/4
  debus_addr/3(out30) -> bram2/1
  debus_din/3(out30) -> bram2/2
  debus_we/3(out30) -> bram2/3
  debus_en/3(out30) -> bram2/4
  debus_addr/4(out29) -> bram3/1
  debus_din/4(out29) -> bram3/2
  debus_we/4(out29) -> bram3/3
  debus_en/4(out29) -> bram3/4
  debus_addr/5(out28) -> bram4/1
  debus_din/5(out28) -> bram4/2
  debus_we/5(out28) -> bram4/3
  debus_en/5(out28) -> bram4/4
  debus_addr/6(out27) -> bram5/1
  debus_din/6(out27) -> bram5/2
  debus_we/6(out27) -> bram5/3
  debus_en/6(out27) -> bram5/4
  debus_addr/7(out26) -> bram6/1
  debus_din/7(out26) -> bram6/2
  debus_we/7(out26) -> bram6/3
  debus_en/7(out26) -> bram6/4
  debus_addr/8(out25) -> bram7/1
  debus_din/8(out25) -> bram7/2
  debus_we/8(out25) -> bram7/3
  debus_en/8(out25) -> bram7/4
  debus_addr/9(out24) -> bram8/1
  debus_din/9(out24) -> bram8/2
  debus_we/9(out24) -> bram8/3
  debus_en/9(out24) -> bram8/4
  debus_addr/10(out23) -> bram9/1
  debus_din/10(out23) -> bram9/2
  debus_we/10(out23) -> bram9/3
  debus_en/10(out23) -> bram9/4
  debus_addr/11(out22) -> bram10/1
  debus_din/11(out22) -> bram10/2
  debus_we/11(out22) -> bram10/3
  debus_en/11(out22) -> bram10/4
  debus_addr/12(out21) -> bram11/1
  debus_din/12(out21) -> bram11/2
  debus_we/12(out21) -> bram11/3
  debus_en/12(out21) -> bram11/4
  debus_addr/13(out20) -> bram12/1
  debus_din/13(out20) -> bram12/2
  debus_we/13(out20) -> bram12/3
  debus_en/13(out20) -> bram12/4
  debus_addr/14(out19) -> bram13/1
  debus_din/14(out19) -> bram13/2
  debus_we/14(out19) -> bram13/3
  debus_en/14(out19) -> bram13/4
  debus_addr/15(out18) -> bram14/1
  debus_din/15(out18) -> bram14/2
  debus_we/15(out18) -> bram14/3
  debus_en/15(out18) -> bram14/4
  debus_addr/16(out17) -> bram15/1
  debus_din/16(out17) -> bram15/2
  debus_we/16(out17) -> bram15/3
  debus_en/16(out17) -> bram15/4
  debus_addr/17(out16) -> bram16/1
  debus_din/17(out16) -> bram16/2
  debus_we/17(out16) -> bram16/3
  debus_en/17(out16) -> bram16/4
  debus_addr/18(out15) -> bram17/1
  debus_din/18(out15) -> bram17/2
  debus_we/18(out15) -> bram17/3
  debus_en/18(out15) -> bram17/4
  debus_addr/19(out14) -> bram18/1
  debus_din/19(out14) -> bram18/2
  debus_we/19(out14) -> bram18/3
  debus_en/19(out14) -> bram18/4
  debus_addr/20(out13) -> bram19/1
  debus_din/20(out13) -> bram19/2
  debus_we/20(out13) -> bram19/3
  debus_en/20(out13) -> bram19/4
  debus_addr/21(out12) -> bram20/1
  debus_din/21(out12) -> bram20/2
  debus_we/21(out12) -> bram20/3
  debus_en/21(out12) -> bram20/4
  debus_addr/22(out11) -> bram21/1
  debus_din/22(out11) -> bram21/2
  debus_we/22(out11) -> bram21/3
  debus_en/22(out11) -> bram21/4
  debus_addr/23(out10) -> bram22/1
  debus_din/23(out10) -> bram22/2
  debus_we/23(out10) -> bram22/3
  debus_en/23(out10) -> bram22/4
  debus_addr/24(out9) -> bram23/1
  debus_din/24(out9) -> bram23/2
  debus_we/24(out9) -> bram23/3
  debus_en/24(out9) -> bram23/4
  debus_addr/25(out8) -> bram24/1
  debus_din/25(out8) -> bram24/2
  debus_we/25(out8) -> bram24/3
  debus_en/25(out8) -> bram24/4
  debus_addr/26(out7) -> bram25/1
  debus_din/26(out7) -> bram25/2
  debus_we/26(out7) -> bram25/3
  debus_en/26(out7) -> bram25/4
  debus_addr/27(out6) -> bram26/1
  debus_din/27(out6) -> bram26/2
  debus_we/27(out6) -> bram26/3
  debus_en/27(out6) -> bram26/4
  debus_addr/28(out5) -> bram27/1
  debus_din/28(out5) -> bram27/2
  debus_we/28(out5) -> bram27/3
  debus_en/28(out5) -> bram27/4
  debus_addr/29(out4) -> bram28/1
  debus_din/29(out4) -> bram28/2
  debus_we/29(out4) -> bram28/3
  debus_en/29(out4) -> bram28/4
  debus_addr/30(out3) -> bram29/1
  debus_din/30(out3) -> bram29/2
  debus_we/30(out3) -> bram29/3
  debus_en/30(out3) -> bram29/4
  debus_addr/31(out2) -> bram30/1
  debus_din/31(out2) -> bram30/2
  debus_we/31(out2) -> bram30/3
  debus_en/31(out2) -> bram30/4
  debus_addr/32(lsb_out1) -> bram31/1
  debus_din/32(lsb_out1) -> bram31/2
  debus_we/32(lsb_out1) -> bram31/3
  debus_en/32(lsb_out1) -> bram31/4, den/1
  bram0/1 -> din_bussify/1(in1)
  bram1/1 -> din_bussify/2(in2)
  bram2/1 -> din_bussify/3(in3)
  bram3/1 -> din_bussify/4(in4)
  bram4/1 -> din_bussify/5(in5)
  bram5/1 -> din_bussify/6(in6)
  bram6/1 -> din_bussify/7(in7)
  bram7/1 -> din_bussify/8(in8)
  bram8/1 -> din_bussify/9(in9)
  bram9/1 -> din_bussify/10(in10)
  bram10/1 -> din_bussify/11(in11)
  bram11/1 -> din_bussify/12(in12)
  bram12/1 -> din_bussify/13(in13)
  bram13/1 -> din_bussify/14(in14)
  bram14/1 -> din_bussify/15(in15)
  bram15/1 -> din_bussify/16(in16)
  bram16/1 -> din_bussify/17(in17)
  bram17/1 -> din_bussify/18(in18)
  bram18/1 -> din_bussify/19(in19)
  bram19/1 -> din_bussify/20(in20)
  bram20/1 -> din_bussify/21(in21)
  bram21/1 -> din_bussify/22(in22)
  bram22/1 -> din_bussify/23(in23)
  bram23/1 -> din_bussify/24(in24)
  bram24/1 -> din_bussify/25(in25)
  bram25/1 -> din_bussify/26(in26)
  bram26/1 -> din_bussify/27(in27)
  bram27/1 -> din_bussify/28(in28)
  bram28/1 -> din_bussify/29(in29)
  bram29/1 -> din_bussify/30(in30)
  bram30/1 -> din_bussify/31(in31)
  bram31/1 -> din_bussify/32(in32)
  din_bussify/1(bus_out) -> dout/1
  den/1 -> dvalid/1

== system r2_c8n856m1k_vacc/pfb/pfb_fir_generic/pfb_fir_taps/en_expand ==
  bus_in : Inport
  slice1 : xbsIndex_r4/Slice  nbits=1  boolean_output=on  mode=Upper Bit Location + Width  bit1=-1  base1=MSB of Input  bit0=0  base0=LSB of Input  dbl_ovrd=off  block_type=slice
  slice2 : xbsIndex_r4/Slice  nbits=1  boolean_output=on  mode=Upper Bit Location + Width  bit1=0  base1=MSB of Input  bit0=0  base0=LSB of Input  dbl_ovrd=off  block_type=slice
  msb_out2 : Outport
  lsb_out1 : Outport  Port=2
  wires:
  bus_in/1 -> slice2/1, slice1/1
  slice2/1 -> msb_out2/1
  slice1/1 -> lsb_out1/1
